simulink model "spec2in_4096ch_500mhz"  (R2013b) (continued)
== system spec2in_4096ch_500mhz == (continued)
      mask help:
      | An explicitly laid-out delay line to avoid mapping to SRL16s
      | for use in pipelining to help achieve timing closure.
      mask init:
      | pipeline_init(gcb,'latency',latency);
      settings: AttributesFormatString=Latency = %<latency>
      mask Display:
      | port_label('input', 1, 'd');
      | port_label('output', 1, 'q');
      | disp(['Z^-', num2str(latency)]);
  pipeline11 : Subsystem  AncestorBlock=casper_library_delays/pipeline  mask(pipeline){latency=1}
      mask help:
      | An explicitly laid-out delay line to avoid mapping to SRL16s
      | for use in pipelining to help achieve timing closure.
      mask init:
      | pipeline_init(gcb,'latency',latency);
      settings: AttributesFormatString=Latency = %<latency>
      mask Display:
      | port_label('input', 1, 'd');
      | port_label('output', 1, 'q');
      | disp(['Z^-', num2str(latency)]);
  pipeline12 : Subsystem  AncestorBlock=casper_library_delays/pipeline  mask(pipeline){latency=1}
      mask help:
      | An explicitly laid-out delay line to avoid mapping to SRL16s
      | for use in pipelining to help achieve timing closure.
      mask init:
      | pipeline_init(gcb,'latency',latency);
      settings: AttributesFormatString=Latency = %<latency>
      mask Display:
      | port_label('input', 1, 'd');
      | port_label('output', 1, 'q');
      | disp(['Z^-', num2str(latency)]);
  pipeline13 : Subsystem  AncestorBlock=casper_library_delays/pipeline  mask(pipeline){latency=1}
      mask help:
      | An explicitly laid-out delay line to avoid mapping to SRL16s
      | for use in pipelining to help achieve timing closure.
      mask init:
      | pipeline_init(gcb,'latency',latency);
      settings: AttributesFormatString=Latency = %<latency>
      mask Display:
      | port_label('input', 1, 'd');
      | port_label('output', 1, 'q');
      | disp(['Z^-', num2str(latency)]);
  pipeline14 : Subsystem  AncestorBlock=casper_library_delays/pipeline  mask(pipeline){latency=1}
      mask help:
      | An explicitly laid-out delay line to avoid mapping to SRL16s
      | for use in pipelining to help achieve timing closure.
      mask init:
      | pipeline_init(gcb,'latency',latency);
      settings: AttributesFormatString=Latency = %<latency>
      mask Display:
      | port_label('input', 1, 'd');
      | port_label('output', 1, 'q');
      | disp(['Z^-', num2str(latency)]);
  pipeline15 : Subsystem  AncestorBlock=casper_library_delays/pipeline  mask(pipeline){latency=1}
      mask help:
      | An explicitly laid-out delay line to avoid mapping to SRL16s
      | for use in pipelining to help achieve timing closure.
      mask init:
      | pipeline_init(gcb,'latency',latency);
      settings: AttributesFormatString=Latency = %<latency>
      mask Display:
      | port_label('input', 1, 'd');
      | port_label('output', 1, 'q');
      | disp(['Z^-', num2str(latency)]);
  pipeline16 : Subsystem  AncestorBlock=casper_library_delays/pipeline  mask(pipeline){latency=1}
      mask help:
      | An explicitly laid-out delay line to avoid mapping to SRL16s
      | for use in pipelining to help achieve timing closure.
      mask init:
      | pipeline_init(gcb,'latency',latency);
      settings: AttributesFormatString=Latency = %<latency>
      mask Display:
      | port_label('input', 1, 'd');
      | port_label('output', 1, 'q');
      | disp(['Z^-', num2str(latency)]);
  pipeline17 : Subsystem  AncestorBlock=casper_library_delays/pipeline  mask(pipeline){latency=1}
      mask help:
      | An explicitly laid-out delay line to avoid mapping to SRL16s
      | for use in pipelining to help achieve timing closure.
      mask init:
      | pipeline_init(gcb,'latency',latency);
      settings: AttributesFormatString=Latency = %<latency>
      mask Display:
      | port_label('input', 1, 'd');
      | port_label('output', 1, 'q');
      | disp(['Z^-', num2str(latency)]);
  pipeline18 : Subsystem  AncestorBlock=casper_library_delays/pipeline  mask(pipeline){latency=1}
      mask help:
      | An explicitly laid-out delay line to avoid mapping to SRL16s
      | for use in pipelining to help achieve timing closure.
      mask init:
      | pipeline_init(gcb,'latency',latency);
      settings: AttributesFormatString=Latency = %<latency>
      mask Display:
      | port_label('input', 1, 'd');
      | port_label('output', 1, 'q');
      | disp(['Z^-', num2str(latency)]);
  pipeline19 : Subsystem  AncestorBlock=casper_library_delays/pipeline  mask(pipeline){latency=1}
      mask help:
      | An explicitly laid-out delay line to avoid mapping to SRL16s
      | for use in pipelining to help achieve timing closure.
      mask init:
      | pipeline_init(gcb,'latency',latency);
      settings: AttributesFormatString=Latency = %<latency>
      mask Display:
      | port_label('input', 1, 'd');
      | port_label('output', 1, 'q');
      | disp(['Z^-', num2str(latency)]);
  pipeline2 : Subsystem  AncestorBlock=casper_library_delays/pipeline  mask(pipeline){latency=1}
      mask help:
      | An explicitly laid-out delay line to avoid mapping to SRL16s
      | for use in pipelining to help achieve timing closure.
      mask init:
      | pipeline_init(gcb,'latency',latency);
      settings: AttributesFormatString=Latency = %<latency>
      mask Display:
      | port_label('input', 1, 'd');
      | port_label('output', 1, 'q');
      | disp(['Z^-', num2str(latency)]);
  pipeline20 : Subsystem  AncestorBlock=casper_library_delays/pipeline  mask(pipeline){latency=1}
      mask help:
      | An explicitly laid-out delay line to avoid mapping to SRL16s
      | for use in pipelining to help achieve timing closure.
      mask init:
      | pipeline_init(gcb,'latency',latency);
      settings: AttributesFormatString=Latency = %<latency>
      mask Display:
      | port_label('input', 1, 'd');
      | port_label('output', 1, 'q');
      | disp(['Z^-', num2str(latency)]);
  pipeline21 : Subsystem  AncestorBlock=casper_library_delays/pipeline  mask(pipeline){latency=2}
      mask help:
      | An explicitly laid-out delay line to avoid mapping to SRL16s
      | for use in pipelining to help achieve timing closure.
      mask init:
      | pipeline_init(gcb,'latency',latency);
      settings: AttributesFormatString=Latency = %<latency>
      mask Display:
      | port_label('input', 1, 'd');
      | port_label('output', 1, 'q');
      | disp(['Z^-', num2str(latency)]);
  pipeline22 : Subsystem  AncestorBlock=casper_library_delays/pipeline  mask(pipeline){latency=1}
      mask help:
      | An explicitly laid-out delay line to avoid mapping to SRL16s
      | for use in pipelining to help achieve timing closure.
      mask init:
      | pipeline_init(gcb,'latency',latency);
      settings: AttributesFormatString=Latency = %<latency>
      mask Display:
      | port_label('input', 1, 'd');
      | port_label('output', 1, 'q');
      | disp(['Z^-', num2str(latency)]);
  pipeline23 : Subsystem  AncestorBlock=casper_library_delays/pipeline  mask(pipeline){latency=1}
      mask help:
      | An explicitly laid-out delay line to avoid mapping to SRL16s
      | for use in pipelining to help achieve timing closure.
      mask init:
      | pipeline_init(gcb,'latency',latency);
      settings: AttributesFormatString=Latency = %<latency>
      mask Display:
      | port_label('input', 1, 'd');
      | port_label('output', 1, 'q');
      | disp(['Z^-', num2str(latency)]);
  pipeline24 : Subsystem  AncestorBlock=casper_library_delays/pipeline  mask(pipeline){latency=1}
      mask help:
      | An explicitly laid-out delay line to avoid mapping to SRL16s
      | for use in pipelining to help achieve timing closure.
      mask init:
      | pipeline_init(gcb,'latency',latency);
      settings: AttributesFormatString=Latency = %<latency>
      mask Display:
      | port_label('input', 1, 'd');
      | port_label('output', 1, 'q');
      | disp(['Z^-', num2str(latency)]);
  pipeline25 : Subsystem  AncestorBlock=casper_library_delays/pipeline  mask(pipeline){latency=1}
      mask help:
      | An explicitly laid-out delay line to avoid mapping to SRL16s
      | for use in pipelining to help achieve timing closure.
      mask init:
      | pipeline_init(gcb,'latency',latency);
      settings: AttributesFormatString=Latency = %<latency>
      mask Display:
      | port_label('input', 1, 'd');
      | port_label('output', 1, 'q');
      | disp(['Z^-', num2str(latency)]);
  pipeline26 : Subsystem  AncestorBlock=casper_library_delays/pipeline  mask(pipeline){latency=1}
      mask help:
      | An explicitly laid-out delay line to avoid mapping to SRL16s
      | for use in pipelining to help achieve timing closure.
      mask init:
      | pipeline_init(gcb,'latency',latency);
      settings: AttributesFormatString=Latency = %<latency>
      mask Display:
      | port_label('input', 1, 'd');
      | port_label('output', 1, 'q');
      | disp(['Z^-', num2str(latency)]);
  pipeline27 : Subsystem  AncestorBlock=casper_library_delays/pipeline  mask(pipeline){latency=1}
      mask help:
      | An explicitly laid-out delay line to avoid mapping to SRL16s
      | for use in pipelining to help achieve timing closure.
      mask init:
      | pipeline_init(gcb,'latency',latency);
      settings: AttributesFormatString=Latency = %<latency>
      mask Display:
      | port_label('input', 1, 'd');
      | port_label('output', 1, 'q');
      | disp(['Z^-', num2str(latency)]);
  pipeline28 : Subsystem  AncestorBlock=casper_library_delays/pipeline  mask(pipeline){latency=1}
      mask help:
      | An explicitly laid-out delay line to avoid mapping to SRL16s
      | for use in pipelining to help achieve timing closure.
      mask init:
      | pipeline_init(gcb,'latency',latency);
      settings: AttributesFormatString=Latency = %<latency>
      mask Display:
      | port_label('input', 1, 'd');
      | port_label('output', 1, 'q');
      | disp(['Z^-', num2str(latency)]);
  pipeline29 : Subsystem  AncestorBlock=casper_library_delays/pipeline  mask(pipeline){latency=1}
      mask help:
      | An explicitly laid-out delay line to avoid mapping to SRL16s
      | for use in pipelining to help achieve timing closure.
      mask init:
      | pipeline_init(gcb,'latency',latency);
      settings: AttributesFormatString=Latency = %<latency>
      mask Display:
      | port_label('input', 1, 'd');
      | port_label('output', 1, 'q');
      | disp(['Z^-', num2str(latency)]);
  pipeline3 : Subsystem  AncestorBlock=casper_library_delays/pipeline  mask(pipeline){latency=1}
      mask help:
      | An explicitly laid-out delay line to avoid mapping to SRL16s
      | for use in pipelining to help achieve timing closure.
      mask init:
      | pipeline_init(gcb,'latency',latency);
      settings: AttributesFormatString=Latency = %<latency>
      mask Display:
      | port_label('input', 1, 'd');
      | port_label('output', 1, 'q');
      | disp(['Z^-', num2str(latency)]);
  pipeline30 : Subsystem  AncestorBlock=casper_library_delays/pipeline  mask(pipeline){latency=1}
      mask help:
      | An explicitly laid-out delay line to avoid mapping to SRL16s
      | for use in pipelining to help achieve timing closure.
      mask init:
      | pipeline_init(gcb,'latency',latency);
      settings: AttributesFormatString=Latency = %<latency>
      mask Display:
      | port_label('input', 1, 'd');
      | port_label('output', 1, 'q');
      | disp(['Z^-', num2str(latency)]);
  pipeline31 : Subsystem  AncestorBlock=casper_library_delays/pipeline  mask(pipeline){latency=1}
      mask help:
      | An explicitly laid-out delay line to avoid mapping to SRL16s
      | for use in pipelining to help achieve timing closure.
      mask init:
      | pipeline_init(gcb,'latency',latency);
      settings: AttributesFormatString=Latency = %<latency>
      mask Display:
      | port_label('input', 1, 'd');
      | port_label('output', 1, 'q');
      | disp(['Z^-', num2str(latency)]);
  pipeline32 : Subsystem  AncestorBlock=casper_library_delays/pipeline  mask(pipeline){latency=1}
      mask help:
      | An explicitly laid-out delay line to avoid mapping to SRL16s
      | for use in pipelining to help achieve timing closure.
      mask init:
      | pipeline_init(gcb,'latency',latency);
      settings: AttributesFormatString=Latency = %<latency>
      mask Display:
      | port_label('input', 1, 'd');
      | port_label('output', 1, 'q');
      | disp(['Z^-', num2str(latency)]);
  pipeline33 : Subsystem  AncestorBlock=casper_library_delays/pipeline  mask(pipeline){latency=1}
      mask help:
      | An explicitly laid-out delay line to avoid mapping to SRL16s
      | for use in pipelining to help achieve timing closure.
      mask init:
      | pipeline_init(gcb,'latency',latency);
      settings: AttributesFormatString=Latency = %<latency>
      mask Display:
      | port_label('input', 1, 'd');
      | port_label('output', 1, 'q');
      | disp(['Z^-', num2str(latency)]);
  pipeline34 : Subsystem  AncestorBlock=casper_library_delays/pipeline  mask(pipeline){latency=1}
      mask help:
      | An explicitly laid-out delay line to avoid mapping to SRL16s
      | for use in pipelining to help achieve timing closure.
      mask init:
      | pipeline_init(gcb,'latency',latency);
      settings: AttributesFormatString=Latency = %<latency>
      mask Display:
      | port_label('input', 1, 'd');
      | port_label('output', 1, 'q');
      | disp(['Z^-', num2str(latency)]);
  pipeline35 : Subsystem  AncestorBlock=casper_library_delays/pipeline  mask(pipeline){latency=1}
      mask help:
      | An explicitly laid-out delay line to avoid mapping to SRL16s
      | for use in pipelining to help achieve timing closure.
      mask init:
      | pipeline_init(gcb,'latency',latency);
      settings: AttributesFormatString=Latency = %<latency>
      mask Display:
      | port_label('input', 1, 'd');
      | port_label('output', 1, 'q');
      | disp(['Z^-', num2str(latency)]);
  pipeline36 : Subsystem  AncestorBlock=casper_library_delays/pipeline  mask(pipeline){latency=2}
      mask help:
      | An explicitly laid-out delay line to avoid mapping to SRL16s
      | for use in pipelining to help achieve timing closure.
      mask init:
      | pipeline_init(gcb,'latency',latency);
      settings: AttributesFormatString=Latency = %<latency>
      mask Display:
      | port_label('input', 1, 'd');
      | port_label('output', 1, 'q');
      | disp(['Z^-', num2str(latency)]);
  pipeline37 : Subsystem  AncestorBlock=casper_library_delays/pipeline  mask(pipeline){latency=1}
      mask help:
      | An explicitly laid-out delay line to avoid mapping to SRL16s
      | for use in pipelining to help achieve timing closure.
      mask init:
      | pipeline_init(gcb,'latency',latency);
      settings: AttributesFormatString=Latency = %<latency>
      mask Display:
      | port_label('input', 1, 'd');
      | port_label('output', 1, 'q');
      | disp(['Z^-', num2str(latency)]);
  pipeline38 : Subsystem  AncestorBlock=casper_library_delays/pipeline  mask(pipeline){latency=1}
      mask help:
      | An explicitly laid-out delay line to avoid mapping to SRL16s
      | for use in pipelining to help achieve timing closure.
      mask init:
      | pipeline_init(gcb,'latency',latency);
      settings: AttributesFormatString=Latency = %<latency>
      mask Display:
      | port_label('input', 1, 'd');
      | port_label('output', 1, 'q');
      | disp(['Z^-', num2str(latency)]);
  pipeline39 : Subsystem  AncestorBlock=casper_library_delays/pipeline  mask(pipeline){latency=1}
      mask help:
      | An explicitly laid-out delay line to avoid mapping to SRL16s
      | for use in pipelining to help achieve timing closure.
      mask init:
      | pipeline_init(gcb,'latency',latency);
      settings: AttributesFormatString=Latency = %<latency>
      mask Display:
      | port_label('input', 1, 'd');
      | port_label('output', 1, 'q');
      | disp(['Z^-', num2str(latency)]);
  pipeline4 : Subsystem  AncestorBlock=casper_library_delays/pipeline  mask(pipeline){latency=1}
      mask help:
      | An explicitly laid-out delay line to avoid mapping to SRL16s
      | for use in pipelining to help achieve timing closure.
      mask init:
      | pipeline_init(gcb,'latency',latency);
      settings: AttributesFormatString=Latency = %<latency>
      mask Display:
      | port_label('input', 1, 'd');
      | port_label('output', 1, 'q');
      | disp(['Z^-', num2str(latency)]);
  pipeline40 : Subsystem  AncestorBlock=casper_library_delays/pipeline  mask(pipeline){latency=1}
      mask help:
      | An explicitly laid-out delay line to avoid mapping to SRL16s
      | for use in pipelining to help achieve timing closure.
      mask init:
      | pipeline_init(gcb,'latency',latency);
      settings: AttributesFormatString=Latency = %<latency>
      mask Display:
      | port_label('input', 1, 'd');
      | port_label('output', 1, 'q');
      | disp(['Z^-', num2str(latency)]);
  pipeline41 : Subsystem  AncestorBlock=casper_library_delays/pipeline  mask(pipeline){latency=1}
      mask help:
      | An explicitly laid-out delay line to avoid mapping to SRL16s
      | for use in pipelining to help achieve timing closure.
      mask init:
      | pipeline_init(gcb,'latency',latency);
      settings: AttributesFormatString=Latency = %<latency>
      mask Display:
      | port_label('input', 1, 'd');
      | port_label('output', 1, 'q');
      | disp(['Z^-', num2str(latency)]);
  pipeline42 : Subsystem  AncestorBlock=casper_library_delays/pipeline  mask(pipeline){latency=2}
      mask help:
      | An explicitly laid-out delay line to avoid mapping to SRL16s
      | for use in pipelining to help achieve timing closure.
      mask init:
      | pipeline_init(gcb,'latency',latency);
      settings: AttributesFormatString=Latency = %<latency>
      mask Display:
      | port_label('input', 1, 'd');
      | port_label('output', 1, 'q');
      | disp(['Z^-', num2str(latency)]);
  pipeline5 : Subsystem  AncestorBlock=casper_library_delays/pipeline  mask(pipeline){latency=2}
      mask help:
      | An explicitly laid-out delay line to avoid mapping to SRL16s
      | for use in pipelining to help achieve timing closure.
      mask init:
      | pipeline_init(gcb,'latency',latency);
      settings: AttributesFormatString=Latency = %<latency>
      mask Display:
      | port_label('input', 1, 'd');
      | port_label('output', 1, 'q');
      | disp(['Z^-', num2str(latency)]);
  pipeline6 : Subsystem  AncestorBlock=casper_library_delays/pipeline  mask(pipeline){latency=2}
      mask help:
      | An explicitly laid-out delay line to avoid mapping to SRL16s
      | for use in pipelining to help achieve timing closure.
      mask init:
      | pipeline_init(gcb,'latency',latency);
      settings: AttributesFormatString=Latency = %<latency>
      mask Display:
      | port_label('input', 1, 'd');
      | port_label('output', 1, 'q');
      | disp(['Z^-', num2str(latency)]);
  pipeline7 : Subsystem  AncestorBlock=casper_library_delays/pipeline  mask(pipeline){latency=1}
      mask help:
      | An explicitly laid-out delay line to avoid mapping to SRL16s
      | for use in pipelining to help achieve timing closure.
      mask init:
      | pipeline_init(gcb,'latency',latency);
      settings: AttributesFormatString=Latency = %<latency>
      mask Display:
      | port_label('input', 1, 'd');
      | port_label('output', 1, 'q');
      | disp(['Z^-', num2str(latency)]);
  pipeline8 : Subsystem  AncestorBlock=casper_library_delays/pipeline  mask(pipeline){latency=1}
      mask help:
      | An explicitly laid-out delay line to avoid mapping to SRL16s
      | for use in pipelining to help achieve timing closure.
      mask init:
      | pipeline_init(gcb,'latency',latency);
      settings: AttributesFormatString=Latency = %<latency>
      mask Display:
      | port_label('input', 1, 'd');
      | port_label('output', 1, 'q');
      | disp(['Z^-', num2str(latency)]);
  pipeline9 : Subsystem  AncestorBlock=casper_library_delays/pipeline  mask(pipeline){latency=1}
      mask help:
      | An explicitly laid-out delay line to avoid mapping to SRL16s
      | for use in pipelining to help achieve timing closure.
      mask init:
      | pipeline_init(gcb,'latency',latency);
      settings: AttributesFormatString=Latency = %<latency>
      mask Display:
      | port_label('input', 1, 'd');
      | port_label('output', 1, 'q');
      | disp(['Z^-', num2str(latency)]);
  power{,1..7} (8 alike) : casper_library_misc/power  BitWidth=18  add_latency=2  mult_latency=3
  simple_bram_vacc0_0 : Subsystem  AncestorBlock=casper_library_accumulators/simple_bram_vacc  mask(simple_bram_vacc){vec_len=1024, arith_type=Unsigned, n_bits=64, bin_pt=35}
      mask prompts: vec_len "Vector length:"; arith_type "Output type:"; n_bits "Bit width (output):"; bin_pt "Binary point (output):"
      mask help: This simple vector accumulator is based on a shift register. It outputs its previous accumulated vector after a new_acc pulse is received.
      mask init:
      | simple_bram_vacc_init(gcb, ...
      |     'vec_len', vec_len, ...
      |     'arith_type', arith_type, ...
      |     'n_bits', n_bits, ...
      |     'bin_pt', bin_pt);
      mask TypeOptions arith_type: Signed | Unsigned
  simple_bram_vacc0_1 : Subsystem  AncestorBlock=casper_library_accumulators/simple_bram_vacc  mask(simple_bram_vacc){vec_len=1024, arith_type=Unsigned, n_bits=64, bin_pt=35}
      mask prompts: vec_len "Vector length:"; arith_type "Output type:"; n_bits "Bit width (output):"; bin_pt "Binary point (output):"
      mask help: This simple vector accumulator is based on a shift register. It outputs its previous accumulated vector after a new_acc pulse is received.
      mask init:
      | simple_bram_vacc_init(gcb, ...
      |     'vec_len', vec_len, ...
      |     'arith_type', arith_type, ...
      |     'n_bits', n_bits, ...
      |     'bin_pt', bin_pt);
      mask TypeOptions arith_type: Signed | Unsigned
  simple_bram_vacc0_2 : Subsystem  AncestorBlock=casper_library_accumulators/simple_bram_vacc  mask(simple_bram_vacc){vec_len=1024, arith_type=Unsigned, n_bits=64, bin_pt=35}
      mask prompts: vec_len "Vector length:"; arith_type "Output type:"; n_bits "Bit width (output):"; bin_pt "Binary point (output):"
      mask help: This simple vector accumulator is based on a shift register. It outputs its previous accumulated vector after a new_acc pulse is received.
      mask init:
      | simple_bram_vacc_init(gcb, ...
      |     'vec_len', vec_len, ...
      |     'arith_type', arith_type, ...
      |     'n_bits', n_bits, ...
      |     'bin_pt', bin_pt);
      mask TypeOptions arith_type: Signed | Unsigned
  simple_bram_vacc0_3 : Subsystem  AncestorBlock=casper_library_accumulators/simple_bram_vacc  mask(simple_bram_vacc){vec_len=1024, arith_type=Unsigned, n_bits=64, bin_pt=35}
      mask prompts: vec_len "Vector length:"; arith_type "Output type:"; n_bits "Bit width (output):"; bin_pt "Binary point (output):"
      mask help: This simple vector accumulator is based on a shift register. It outputs its previous accumulated vector after a new_acc pulse is received.
      mask init:
      | simple_bram_vacc_init(gcb, ...
      |     'vec_len', vec_len, ...
      |     'arith_type', arith_type, ...
      |     'n_bits', n_bits, ...
      |     'bin_pt', bin_pt);
      mask TypeOptions arith_type: Signed | Unsigned
  simple_bram_vacc1_0 : Subsystem  AncestorBlock=casper_library_accumulators/simple_bram_vacc  mask(simple_bram_vacc){vec_len=1024, arith_type=Unsigned, n_bits=64, bin_pt=35}
      mask prompts: vec_len "Vector length:"; arith_type "Output type:"; n_bits "Bit width (output):"; bin_pt "Binary point (output):"
      mask help: This simple vector accumulator is based on a shift register. It outputs its previous accumulated vector after a new_acc pulse is received.
      mask init:
      | simple_bram_vacc_init(gcb, ...
      |     'vec_len', vec_len, ...
      |     'arith_type', arith_type, ...
      |     'n_bits', n_bits, ...
      |     'bin_pt', bin_pt);
      mask TypeOptions arith_type: Signed | Unsigned
  simple_bram_vacc1_1 : Subsystem  AncestorBlock=casper_library_accumulators/simple_bram_vacc  mask(simple_bram_vacc){vec_len=1024, arith_type=Unsigned, n_bits=64, bin_pt=35}
      mask prompts: vec_len "Vector length:"; arith_type "Output type:"; n_bits "Bit width (output):"; bin_pt "Binary point (output):"
      mask help: This simple vector accumulator is based on a shift register. It outputs its previous accumulated vector after a new_acc pulse is received.
      mask init:
      | simple_bram_vacc_init(gcb, ...
      |     'vec_len', vec_len, ...
      |     'arith_type', arith_type, ...
      |     'n_bits', n_bits, ...
      |     'bin_pt', bin_pt);
      mask TypeOptions arith_type: Signed | Unsigned
  simple_bram_vacc1_2 : Subsystem  AncestorBlock=casper_library_accumulators/simple_bram_vacc  mask(simple_bram_vacc){vec_len=1024, arith_type=Unsigned, n_bits=64, bin_pt=35}
      mask prompts: vec_len "Vector length:"; arith_type "Output type:"; n_bits "Bit width (output):"; bin_pt "Binary point (output):"
      mask help: This simple vector accumulator is based on a shift register. It outputs its previous accumulated vector after a new_acc pulse is received.
      mask init:
      | simple_bram_vacc_init(gcb, ...
      |     'vec_len', vec_len, ...
      |     'arith_type', arith_type, ...
      |     'n_bits', n_bits, ...
      |     'bin_pt', bin_pt);
      mask TypeOptions arith_type: Signed | Unsigned
  simple_bram_vacc1_3 : Subsystem  AncestorBlock=casper_library_accumulators/simple_bram_vacc  mask(simple_bram_vacc){vec_len=1024, arith_type=Unsigned, n_bits=64, bin_pt=35}
      mask prompts: vec_len "Vector length:"; arith_type "Output type:"; n_bits "Bit width (output):"; bin_pt "Binary point (output):"
      mask help: This simple vector accumulator is based on a shift register. It outputs its previous accumulated vector after a new_acc pulse is received.
      mask init:
      | simple_bram_vacc_init(gcb, ...
      |     'vec_len', vec_len, ...
      |     'arith_type', arith_type, ...
      |     'n_bits', n_bits, ...
      |     'bin_pt', bin_pt);
      mask TypeOptions arith_type: Signed | Unsigned
  sync_gen : Subsystem  AncestorBlock=casper_library_misc/sync_gen  mask(sync_gen){sim_acc_len=1, fft_size=8192, fft_simult_inputs=8, pfb_fir_taps=4, reorder_vec=[2 2 5], scale=1}
      mask prompts: sim_acc_len "Simulation Accumulation Length"; fft_size "FFT Size"; fft_simult_inputs "Simultaneous Inputs (FFT)"; pfb_fir_taps "Taps in pfb_fir (use 1 if no pfb_fir)"; reorder_vec "Reorder Orders (e.g. [2 2 2])"
      mask help:
      | This block computes the sync period based on system parameters as
      | described in Casper memo.  Detailed descritions of the block's
      | variables can be found in the 'Block Documentation' section of
      | the CASPER wiki.
      mask init:
      | sync_gen_init(gcb, ...
      |     'sim_acc_len', sim_acc_len, ...
      |     'fft_size', fft_size, ...
      |     'fft_simult_inputs', fft_simult_inputs,...
      |     'pfb_fir_taps', pfb_fir_taps, ...
      |     'reorder_vec', reorder_vec, ...
      |     'scale', scale);
      settings: AttributesFormatString=sim_sync_period=40960
      mask Help:
      | SyncPeriod=LCM(reorder_vec)*(acc_num)*(pfb_fir_len)*(fft_size/fft_simult_inputs)
      | 
      | See CASPER sync pulse memo for more details.
  wires:
  dout0_0/1(data_out) -> Terminator/1
  dout0_1/1(data_out) -> Terminator1/1
  dout0_2/1(data_out) -> Terminator2/1
  dout0_3/1(data_out) -> Terminator3/1
  Reinterpret/1 -> dout0_0/2(data_in)
  Reinterpret1/1 -> dout0_1/2(data_in)
  Reinterpret2/1 -> dout0_2/2(data_in)
  Reinterpret3/1 -> dout0_3/2(data_in)
  Counter/1 -> dout0_1/1(addr), dout0_3/1(addr), dout0_2/1(addr), dout0_0/1(addr)
  simple_bram_vacc0_0/1(dout) -> Reinterpret/1
  simple_bram_vacc0_0/2(valid) -> Counter/2, dout0_0/3(we)
  simple_bram_vacc0_1/2(valid) -> dout0_1/3(we)
  simple_bram_vacc0_1/1(dout) -> Reinterpret1/1
  simple_bram_vacc0_2/2(valid) -> dout0_2/3(we)
  simple_bram_vacc0_2/1(dout) -> Reinterpret2/1
  simple_bram_vacc0_3/1(dout) -> Reinterpret3/1
  simple_bram_vacc0_3/2(valid) -> dout0_3/3(we)
  acc_cntrl0/1(new_acc) -> pipeline/1(d)
  pipeline/1(q) -> simple_bram_vacc0_1/1(new_acc), simple_bram_vacc0_3/1(new_acc), simple_bram_vacc0_2/1(new_acc), simple_bram_vacc0_0/1(new_acc), Counter/1
  pipeline1/1(q) -> simple_bram_vacc0_0/2(din)
  pipeline2/1(q) -> simple_bram_vacc0_1/2(din)
  pipeline3/1(q) -> simple_bram_vacc0_2/2(din)
  pipeline4/1(q) -> simple_bram_vacc0_3/2(din)
  cnt_rst/1(in_reg) -> pipeline5/1(d)
  pipeline5/1(q) -> Slice/1
  Slice/1 -> [cnt_rst]
  acc_len/1(in_reg) -> pipeline6/1(d)
  pipeline6/1(q) -> [acc_len]
  [acc_len] -> acc_cntrl0/1(acc_len)
  [cnt_rst] -> acc_cntrl0/3(rst)
  Delay/1 -> pipeline1/1(d)
  Delay1/1 -> pipeline2/1(d)
  Delay2/1 -> pipeline3/1(d)
  Delay3/1 -> pipeline4/1(d)
  pipeline10/1(q) -> Delay/1
  pipeline9/1(q) -> Delay1/1
  pipeline8/1(q) -> Delay2/1
  pipeline7/1(q) -> Delay3/1
  power/1 -> pipeline10/1(d)
  power1/1 -> pipeline9/1(d)
  power2/1 -> pipeline8/1(d)
  power3/1 -> pipeline7/1(d)
  pipeline11/1(q) -> acc_cntrl0/2(sync)
  Delay7/1 -> power3/1
  Delay6/1 -> power2/1
  Delay5/1 -> power1/1
  Delay4/1 -> power/1
  Delay8/1 -> pipeline11/1(d)
  Delay13/1 -> Delay7/1
  Delay12/1 -> Delay6/1
  Delay11/1 -> Delay5/1
  Delay10/1 -> Delay4/1
  Delay14/1 -> Delay9/1
  Delay9/1 -> Delay8/1
  fft_wideband_real0/2(out00) -> Delay10/1
  fft_wideband_real0/3(out01) -> Delay11/1
  fft_wideband_real0/4(out02) -> Delay12/1
  fft_wideband_real0/5(out03) -> Delay13/1
  fft_wideband_real0/1(sync_out) -> Delay14/1
  fft_wideband_real0/6(of) -> Terminator4/1
  Constant2/1 -> fft_wideband_real0/2(shift)
  Delay15/1 -> fft_wideband_real0/1(sync)
  Shift/1 -> fft_wideband_real0/3(in00)
  Shift1/1 -> fft_wideband_real0/4(in01)
  Shift2/1 -> fft_wideband_real0/5(in02)
  Shift3/1 -> fft_wideband_real0/6(in03)
  Shift4/1 -> fft_wideband_real0/7(in04)
  Shift5/1 -> fft_wideband_real0/8(in05)
  Shift6/1 -> fft_wideband_real0/9(in06)
  Shift7/1 -> fft_wideband_real0/10(in07)
  pfb_fir_real0/1(sync_out) -> Delay15/1
  pfb_fir_real0/2(pol1_out1) -> Shift/1
  pfb_fir_real0/3(pol1_out2) -> Shift1/1
  pfb_fir_real0/4(pol1_out3) -> Shift2/1
  pfb_fir_real0/5(pol1_out4) -> Shift3/1
  pfb_fir_real0/6(pol1_out5) -> Shift4/1
  pfb_fir_real0/7(pol1_out6) -> Shift5/1
  pfb_fir_real0/8(pol1_out7) -> Shift6/1
  pfb_fir_real0/9(pol1_out8) -> Shift7/1
  pipeline20/1(q) -> pfb_fir_real0/1(sync)
  pipeline19/1(q) -> pfb_fir_real0/2(pol1_in1)
  pipeline18/1(q) -> pfb_fir_real0/3(pol1_in2)
  pipeline17/1(q) -> pfb_fir_real0/4(pol1_in3)
  pipeline16/1(q) -> pfb_fir_real0/5(pol1_in4)
  pipeline15/1(q) -> pfb_fir_real0/6(pol1_in5)
  pipeline14/1(q) -> pfb_fir_real0/7(pol1_in6)
  pipeline13/1(q) -> pfb_fir_real0/8(pol1_in7)
  pipeline12/1(q) -> pfb_fir_real0/9(pol1_in8)
  adc083000x2/1(adc0_s0) -> internal_oor0/1(In1), bus_create0/1(in1), pipeline19/1(d)
  adc083000x2/2(adc0_s1) -> pipeline18/1(d), internal_oor0/2(In2), bus_create0/2(in2)
  adc083000x2/3(adc0_s2) -> internal_oor0/3(In3), bus_create0/3(in3), pipeline17/1(d)
  adc083000x2/4(adc0_s3) -> pipeline16/1(d), internal_oor0/4(In4), bus_create0/4(in4)
  adc083000x2/5(adc0_s4) -> internal_oor0/5(In5), bus_create0/5(in5), pipeline15/1(d)
  adc083000x2/6(adc0_s5) -> internal_oor0/6(In6), bus_create0/6(in6), pipeline14/1(d)
  adc083000x2/7(adc0_s6) -> pipeline13/1(d), internal_oor0/7(In7), bus_create0/7(in7)
  adc083000x2/8(adc0_s7) -> internal_oor0/8(In8), bus_create0/8(in8), pipeline12/1(d)
  [sync_gen] -> pipeline20/1(d)
  bus_create0/1(bus_out) -> pipeline21/1(d)
  Constant5/1 -> adcsnap0/2(we), adcsnap0/3(trig)
  pipeline21/1(q) -> adcsnap0/1(din)
  [cnt_rst] -> internal_oor0/9(rst)
  internal_oor0/1(out_of_range) -> led_0/1
  led_0/1 -> Terminator8/1
  dout1_0/1(data_out) -> Terminator9/1
  dout1_1/1(data_out) -> Terminator10/1
  dout1_2/1(data_out) -> Terminator11/1
  dout1_3/1(data_out) -> Terminator12/1
  Reinterpret4/1 -> dout1_0/2(data_in)
  Reinterpret5/1 -> dout1_1/2(data_in)
  Reinterpret6/1 -> dout1_2/2(data_in)
  Reinterpret7/1 -> dout1_3/2(data_in)
  Counter1/1 -> dout1_1/1(addr), dout1_3/1(addr), dout1_2/1(addr), dout1_0/1(addr)
  simple_bram_vacc1_0/1(dout) -> Reinterpret4/1
  simple_bram_vacc1_0/2(valid) -> Counter1/2, dout1_0/3(we)
  simple_bram_vacc1_1/2(valid) -> dout1_1/3(we)
  simple_bram_vacc1_1/1(dout) -> Reinterpret5/1
  simple_bram_vacc1_2/2(valid) -> dout1_2/3(we)
  simple_bram_vacc1_2/1(dout) -> Reinterpret6/1
  simple_bram_vacc1_3/1(dout) -> Reinterpret7/1
  simple_bram_vacc1_3/2(valid) -> dout1_3/3(we)
  acc_cntrl1/1(new_acc) -> pipeline22/1(d)
  pipeline22/1(q) -> simple_bram_vacc1_1/1(new_acc), simple_bram_vacc1_3/1(new_acc), simple_bram_vacc1_2/1(new_acc), simple_bram_vacc1_0/1(new_acc), Counter1/1
  pipeline23/1(q) -> simple_bram_vacc1_0/2(din)
  pipeline34/1(q) -> simple_bram_vacc1_1/2(din)
  pipeline37/1(q) -> simple_bram_vacc1_2/2(din)
  pipeline38/1(q) -> simple_bram_vacc1_3/2(din)
  [acc_len] -> acc_cntrl1/1(acc_len)
  [cnt_rst] -> acc_cntrl1/3(rst)
  Delay17/1 -> pipeline23/1(d)
  Delay18/1 -> pipeline34/1(d)
  Delay26/1 -> pipeline37/1(d)
  Delay27/1 -> pipeline38/1(d)
  pipeline24/1(q) -> Delay17/1
  pipeline41/1(q) -> Delay18/1
  pipeline40/1(q) -> Delay26/1
  pipeline39/1(q) -> Delay27/1
  power4/1 -> pipeline24/1(d)
  power5/1 -> pipeline41/1(d)
  power6/1 -> pipeline40/1(d)
  power7/1 -> pipeline39/1(d)
  pipeline25/1(q) -> acc_cntrl1/2(sync)
  Delay31/1 -> power7/1
  Delay30/1 -> power6/1
  Delay29/1 -> power5/1
  Delay28/1 -> power4/1
  Delay32/1 -> pipeline25/1(d)
  Delay22/1 -> Delay31/1
  Delay21/1 -> Delay30/1
  Delay20/1 -> Delay29/1
  Delay19/1 -> Delay28/1
  Delay23/1 -> Delay33/1
  Delay33/1 -> Delay32/1
  fft_wideband_real1/2(out00) -> Delay19/1
  fft_wideband_real1/3(out01) -> Delay20/1
  fft_wideband_real1/4(out02) -> Delay21/1
  fft_wideband_real1/5(out03) -> Delay22/1
  fft_wideband_real1/1(sync_out) -> Delay23/1
  fft_wideband_real1/6(of) -> Terminator13/1
  Constant6/1 -> fft_wideband_real1/2(shift)
  Delay24/1 -> fft_wideband_real1/1(sync)
  Shift8/1 -> fft_wideband_real1/3(in00)
  Shift9/1 -> fft_wideband_real1/4(in01)
  Shift10/1 -> fft_wideband_real1/5(in02)
  Shift11/1 -> fft_wideband_real1/6(in03)
  Shift12/1 -> fft_wideband_real1/7(in04)
  Shift13/1 -> fft_wideband_real1/8(in05)
  Shift14/1 -> fft_wideband_real1/9(in06)
  Shift15/1 -> fft_wideband_real1/10(in07)
  pfb_fir_real1/1(sync_out) -> Delay24/1
  pfb_fir_real1/2(pol1_out1) -> Shift8/1
  pfb_fir_real1/3(pol1_out2) -> Shift9/1
  pfb_fir_real1/4(pol1_out3) -> Shift10/1
  pfb_fir_real1/5(pol1_out4) -> Shift11/1
  pfb_fir_real1/6(pol1_out5) -> Shift12/1
  pfb_fir_real1/7(pol1_out6) -> Shift13/1
  pfb_fir_real1/8(pol1_out7) -> Shift14/1
  pfb_fir_real1/9(pol1_out8) -> Shift15/1
  pipeline35/1(q) -> pfb_fir_real1/1(sync)
  pipeline33/1(q) -> pfb_fir_real1/2(pol1_in1)
  pipeline32/1(q) -> pfb_fir_real1/3(pol1_in2)
  pipeline31/1(q) -> pfb_fir_real1/4(pol1_in3)
  pipeline30/1(q) -> pfb_fir_real1/5(pol1_in4)
  pipeline29/1(q) -> pfb_fir_real1/6(pol1_in5)
  pipeline28/1(q) -> pfb_fir_real1/7(pol1_in6)
  pipeline27/1(q) -> pfb_fir_real1/8(pol1_in7)
  pipeline26/1(q) -> pfb_fir_real1/9(pol1_in8)
  adc083000x2/12(adc1_s0) -> internal_oor1/1(In1), bus_create1/1(in1), pipeline33/1(d)
  adc083000x2/13(adc1_s1) -> internal_oor1/2(In2), bus_create1/2(in2), pipeline32/1(d)
  adc083000x2/14(adc1_s2) -> internal_oor1/3(In3), bus_create1/3(in3), pipeline31/1(d)
  adc083000x2/15(adc1_s3) -> internal_oor1/4(In4), bus_create1/4(in4), pipeline30/1(d)
  adc083000x2/16(adc1_s4) -> internal_oor1/5(In5), bus_create1/5(in5), pipeline29/1(d)
  adc083000x2/17(adc1_s5) -> internal_oor1/6(In6), bus_create1/6(in6), pipeline28/1(d)
  adc083000x2/18(adc1_s6) -> internal_oor1/7(In7), bus_create1/7(in7), pipeline27/1(d)
  adc083000x2/19(adc1_s7) -> internal_oor1/8(In8), bus_create1/8(in8), pipeline26/1(d)
  [sync_gen] -> pipeline35/1(d)
  bus_create1/1(bus_out) -> pipeline36/1(d)
  Constant9/1 -> adcsnap1/2(we), adcsnap1/3(trig)
  pipeline36/1(q) -> adcsnap1/1(din)
  [cnt_rst] -> internal_oor1/9(rst)
  internal_oor1/1(out_of_range) -> led_1/1
  led_1/1 -> Terminator17/1
  adc083000x2/22(adc1_data_valid) -> Terminator7/1
  adc083000x2/21(adc1_outofrange) -> Terminator6/1
  adc083000x2/20(adc1_sync) -> Terminator5/1
  adc083000x2/11(adc0_data_valid) -> Terminator16/1
  adc083000x2/10(adc0_outofrange) -> Terminator15/1
  adc083000x2/9(adc0_sync) -> Terminator14/1
  Constant3/1 -> adc083000x2/1(adc0_sync_sim)
  Constant4/1 -> adc083000x2/2(adc0_data_valid_sim)
  Band-Limited White Noise/1 -> adc083000x2/5(sim_adc0_data_in)
  Band-Limited White Noise1/1 -> adc083000x2/6(sim_adc1_data_in)
  Constant7/1 -> adc083000x2/3(adc1_sync_sim)
  Constant8/1 -> adc083000x2/4(adc1_data_valid_sim)
  sync_gen/1(sync_out) -> pipeline42/1(d)
  pipeline42/1(q) -> [sync_gen]
